simulink model "vegas01_4tap_1024ch_250mhz_r1"  (Simulink 7.6)
settings: Solver=ode45  StartTime=0.0  StopTime=128000  RelTol=1e-3
undefined symbols (not defined in the file): ROACH
libraries: xbsIndex_r4 x12350, monroe_library x84, xbsReplacements_r4 x36, xps_library x30, casper_library_misc x24, dspsigops x16
(part 9 of 103)

== system vegas01_4tap_1024ch_250mhz_r1/pfb_fir_real2/coeff_gen_2_5 ==
  sync : Inport
  Counter0 : xbsIndex_r4/Counter  cnt_type=Free Running  cnt_to=Inf  operation=Up  start_count=0  cnt_by_val=1  arith_type=Unsigned  n_bits=8  bin_pt=0  load_pin=off  rst=on  en=off  explicit_period=on  period=1  dbl_ovrd=off  use_behavioral_HDL=off  implementation=Fabric  block_type=counter
  Counter1 : xbsIndex_r4/Counter  cnt_type=Free Running  cnt_to=Inf  operation=Down  start_count=255  cnt_by_val=1  arith_type=Unsigned  n_bits=8  bin_pt=0  load_pin=off  rst=on  en=off  explicit_period=on  period=1  dbl_ovrd=off  use_behavioral_HDL=off  implementation=Fabric  block_type=counter
  coeff_ram_1 : xbsIndex_r4/Dual Port RAM  depth=256  initVector=[-3.90817859526566e-05 -0.00019581594613078 -0.000353262922406334 -0.000511505297702878 -0.000670626183556419 -0.000830709171084608 -0.00099183828144884 -0.00115409791582477 -0.00131757280489769 -0.00148234795789864 -0.00164850861119816 -0.00181614017647413 -0.00198532818847055 -0.00215615825236462 -0.00232871599075906 -0.00250308699031737 -0.00267935674805954 -0.00285761061733611 -0.00303793375349835 -0.003220411059283 -0.00340512712992955 -0.0035921661980487 -0.00378161207826056 -0.00397354811162116 -0.00416805710985634 -0.00436522129942181 -0.00456512226540876 -0.00476784089531378 -0.00497345732269304 -0.00518205087071952 -0.00539369999566348 -0.0056084822303152 -0.00582647412737009 -0.00604775120279601 -0.00627238787920226 -0.00650045742923075 -0.00673203191898883 -0.00696718215154433 -0.00720597761050248 -0.00744848640368526 -0.00769477520693305 -0.00794490920804915 -0.00819895205090707 -0.00845696577974117 -0.0087190107836408 -0.0089851457412682 -0.0092554275658206 -0.00952991135025664 -0.00980865031280764 -0.0100916957427936 -0.0103790969467645 -0.0106709011949873 -0.0109671536682976 -0.0112678974053384 -0.011573173250203 -0.011883019800505 -0.0121974733558934 -0.0125165678670334 -0.0128403348850725 -0.0131688035116118 -0.0135020003492023 -0.0138399494523852 -0.0141826722792961 -0.0145301876438517 -0.0148825116685399 -0.0152396577378301 -0.0156016364522244 -0.0159684555829678 -0.0163401200274364 -0.0167166317652216 -0.0170979898149293 -0.0174841901917123 -0.017875225865553 -0.0182710867203162 -0.0186717595135873 -0.0190772278373159 -0.0194874720792798 -0.0199024693853888 -0.0203221936228435 -0.0207466153441663 -0.0211757017521223 -0.0216094166655443 -0.0220477204860799 -0.0224905701658759 -0.0229379191762147 -0.02338971747712 -0.0238459114879449 -0.0243064440589593 -0.0247712544439492 -0.025240278273844 -0.0257134475313849 -0.0261906905268485 -0.0266719318748394 -0.0271570924721649 -0.0276460894768045 -0.0281388362879872 -0.0286352425273895 -0.0291352140214652 -0.0296386527849192 -0.0301454570053374 -0.030655521028983 -0.0311687353477706 -0.031684986587428 -0.0322041574968574 -0.0327261269387038 -0.0332507698811416 -0.0337779573908884 -0.0343075566274544 -0.0348394308386364 -0.0353734393572641 -0.0359094375992071 -0.0364472770626494 -0.0369868053286391 -0.0375278660629194 -0.0380702990190484 -0.0386139400428121 -0.0391586210779373 -0.0397041701731098 -0.040250411490302 -0.0407971653144147 -0.041344248064238 -0.0418914723047336 -0.0424386467606426 -0.0429855763314227 -0.0435320621075147 -0.044077901387944 -0.0446228876992558 -0.045166810815787 -0.0457094567812755 -0.0462506079318073 -0.0467900429201015 -0.0473275367411334 -0.0478628607590946 -0.0483957827356896 -0.0489260668597665 -0.0494534737782813 -0.0499777606285926 -0.050498681072084 -0.051015985329112 -0.051529420215275 -0.052038729179 -0.0525436523404422 -0.0530439265316944 -0.0535392853382986 -0.0540294591420561 -0.0545141751651301 -0.0549931575154334 -0.055466127233296 -0.0559328023394035 -0.0563928978840011 -0.0568461259973533 -0.0572921959414519 -0.0577308141629635 -0.0581616843474073 -0.0585845074745537 -0.058998981875033 -0.0594048032881459 -0.0598016649208627 -0.0601892575080014 -0.0605672693735733 -0.060935386493284 -0.0612932925581768 -0.0616406690394071 -0.0619771952541345 -0.0623025484325178 -0.0626164037858017 -0.0629184345754786 -0.0632083121835126 -0.0634857061836099 -0.0637502844135214 -0.0640017130483604 -0.0642396566749216 -0.0644637783669827 -0.064673739761574 -0.0648692011361979 -0.0650498214869818 -0.0652152586077456 -0.0653651691699669 -0.0654992088036259 -0.0656170321789096 -0.0657182930887587 -0.065802644532236 -0.0658697387986982 -0.0659192275527504 -0.0659507619199633 -0.0659639925733332 -0.0659585698204637 -0.0659341436914474 -0.0658903640274281 -0.0658268805698202 -0.0657433430501645 -0.0656394012805979 -0.0655147052449153 -0.0653689051901997 -0.0652016517189997 -0.0650125958820299 -0.0648013892713714 -0.0645676841141486 ...(+1158 chars)]  distributed_mem=Block RAM  init_a=0  init_b=0  rst_a=off  rst_b=off  en_a=off  en_b=off  latency=3  write_mode_A=No Read On Write  write_mode_B=No Read On Write  dbl_ovrd=off  optimize=Area  use_rpm=on  block_type=dpram
  coeff_ram_2 : xbsIndex_r4/Dual Port RAM  depth=256  initVector=[0.000528634489950224 0.00266729854995164 0.00484466034859744 0.00706084272688782 0.0093159621512389 0.0116101286431996 0.0139434457103661 0.0163160102785174 0.018727912624994 0.0211792363133421 0.0236700581292446 0.0262004480177607 0.0287704690218949 0.0313801772225176 0.0340296216796558 0.036718844375176 0.0394478801568792 0.0422167566840262 0.0450254943743139 0.0478741063523217 0.0507625983994457 0.0536909689053397 0.0566592088208814 0.0596673016126801 0.0627152232191451 0.0658029420081291 0.0689304187361667 0.0720976065093205 0.0753044507456532 0.0785508891393394 0.0818368516264341 0.0851622603523098 0.0885270296407792 0.0919310659649154 0.0953742679195836 0.0988565261956976 0.102377723556213 0.10593773481387 0.109536426810698 0.11317365839929 0.116849280425861 0.120563135715103 0.124315059056838 0.128104877194488 0.131932408815367 0.1357974645428 0.139699846930085 0.143639350456298 0.147615761523955 0.151628858458532 0.155678411509854 0.159764182855352 0.163885926605205 0.168043388809357 0.172236307466429 0.176464412534516 0.180727425943882 0.185025061611548 0.18935702545779 0.193723015424524 0.198122721495609 0.202555825719045 0.207022002231081 0.211520917282222 0.216052229265149 0.220615588744533 0.225210638488761 0.22983701350355 0.234494341067471 0.23918224076936 0.243900324547619 0.248648196731411 0.253425454083732 0.258231685846359 0.263066473786681 0.267929392246381 0.272820008191991 0.277737881267295 0.282682563847572 0.287653601095688 0.292650531020009 0.297672884534137 0.302720185518453 0.307791950883472 0.312887690634974 0.318006907940928 0.323149099200174 0.328313754112871 0.333500355752685 0.338708380640704 0.343937298821085 0.34918657393839 0.354455663316627 0.359744018039958 0.365051083035069 0.370376297155193 0.375719093265751 0.38107889833162 0.386455133505983 0.391847214220772 0.39725455027866 0.402676545946607 0.408112600050922 0.413562106073836 0.419024452251563 0.424499021673817 0.429985192384788 0.435482337485536 0.440989825237791 0.446507019169137 0.452033278179554 0.457567956649303 0.463110404548126 0.468659967545736 0.474215987123581 0.479777800687855 0.485344741683723 0.490916139710754 0.496491320639523 0.502069606729355 0.507650316747201 0.513232766087606 0.518816266893743 0.524400128179501 0.52998365595258 0.535566153338583 0.54114692070607 0.546725255792543 0.552300453831348 0.557871807679439 0.563438607946017 0.569000143121969 0.574555699710111 0.580104562356196 0.585646013980653 0.591179335911029 0.596703808015113 0.6022187088347 0.607723315719968 0.613216904964448 0.618698751940534 0.624168131235529 0.629624316788173 0.63506658202564 0.640494200000953 0.645906443530805 0.651302585333747 0.656681898168698 0.662043654973776 0.667387129005377 0.672711593977511 0.678016324201334 0.68330059472485 0.688563681472764 0.693804861386435 0.699023412563906 0.70421861439998 0.709389747726301 0.714536094951418 0.719656940200789 0.724751569456699 0.729819270698056 0.734859334040036 0.739871051873534 0.744853719004404 0.74980663279244 0.754729093290075 0.759620403380761 0.764479868916999 0.76930679885798 0.774100505406823 0.778860304147345 0.78358551418037 0.788275458259508 0.7929294629264 0.797546858645375 0.802126979937506 0.806669165514013 0.811172758409008 0.815637106111515 0.820061560696764 0.82444547895672 0.828788222529799 0.833089158029764 0.837347657173754 0.841563096909419 0.845734859541138 0.849862332855275 0.853944910244456 0.857981990830835 0.861972979588306 0.865917287463656 0.869814331496604 0.873663534938716 0.877464327371157 0.881216144821258 0.88491842987786 0.88857063180542 0.892172206656845 0.895722617385021 0.89922133395302 0.902667833442954 0.90606160016345 0.909402125755714 0.912688909298172 0.915921457409648 0.919099284351063 0.922221912125626 0.925288870577496 0.928299697488889 0.931253938675603 0.934151148080945 0.936990887868035 0.939772728510454 0.942496248881234 0.945161036340149 0.947766686819299 0.950312804906955 0.952799003929657 0.955224906032532 0.957590142257825 ...(+626 chars)]  distributed_mem=Block RAM  init_a=0  init_b=0  rst_a=off  rst_b=off  en_a=off  en_b=off  latency=3  write_mode_A=No Read On Write  write_mode_B=No Read On Write  dbl_ovrd=off  optimize=Area  use_rpm=on  block_type=dpram
  coeff_ram_3 : xbsIndex_r4/Dual Port RAM  depth=256  initVector=[0.999997585420204 0.999945862035735 0.999826625478213 0.999639894159387 0.999385696890974 0.999064072878614 0.998675071713642 0.99821875336269 0.997695188155105 0.997104456768201 0.996446650210344 0.99572186980187 0.994930227153842 0.99407184414466 0.993146852894515 0.992155395737707 0.991097625192822 0.98997370393079 0.988783804740809 0.987528110494175 0.986206814105993 0.984820118494805 0.983368236540129 0.98185139103793 0.980269814654024 0.978623749875434 0.976913448959704 0.975139173882195 0.973301196281355 0.971399797402003 0.969435268036615 0.967407908464641 0.965318028389877 0.963165946875873 0.960951992279441 0.958676502182232 0.956339823320431 0.953942311512578 0.951484331585526 0.94896625729856 0.946388471265699 0.943751364876193 0.941055338213235 0.938300799970914 0.935488167369428 0.932617866068564 0.929690330079492 0.926706001674867 0.923665331297283 0.920568777466085 0.917416806682576 0.914209893333624 0.91094851959372 0.907633175325473 0.904264357978609 0.900842572487458 0.897368331166986 0.89384215360738 0.89026456656721 0.88663610386521 0.882957306270688 0.879228721392593 0.875450903567282 0.87162441374499 0.867749819375054 0.863827694289899 0.859858618587827 0.855843178514634 0.851781966344081 0.847675580257249 0.843524624220809 0.839329707864236 0.835091446355994 0.830810460278722 0.826487375503459 0.822122823062921 0.817717439023884 0.81327186435868 0.808786744815852 0.80426273079 0.799700477190831 0.795100643311471 0.79046389269605 0.785790893006594 0.781082315889274 0.776338836840015 0.77156113506952 0.766749893367735 0.761905797967781 0.75702953840939 0.752121807401883 0.74718330068671 0.742214716899598 0.737216757432325 0.732190126294173 0.727135529973066 0.722053677296456 0.71694527929196 0.711811049047805 0.706651701573101 0.701467953657975 0.696260523733609 0.691030131732197 0.685777498946876 0.680503347891644 0.675208402161309 0.669893386291496 0.664559025618754 0.65920604614078 0.653835174376808 0.648447137228184 0.643042661839171 0.637622475457997 0.632187305298207 0.626737878400318 0.621274921493837 0.615799160859656 0.610311322192862 0.604812130466 0.5993023097928 0.593782583292427 0.588253672954258 0.582716299503241 0.577171182265841 0.571619039036634 0.566060585945546 0.560496537325794 0.554927605582544 0.549354501062321 0.543777931923198 0.538198604005794 0.532617220705113 0.527034482843242 0.52145108854295 0.515867733102212 0.510285108869672 0.504703905121097 0.499124807936828 0.493548500080263 0.487975660877405 0.482406966097486 0.476843087834706 0.47128469439111 0.465732450160622 0.460187015514271 0.454649046686618 0.449119195663434 0.443598110070618 0.438086433064416 0.432584803222934 0.42709385443898 0.421614215814265 0.41614651155497 0.410691360868709 0.405249377862908 0.399821171444622 0.394407345221811 0.389008497406085 0.383625220716957 0.378258102287602 0.37290772357216 0.367574660254585 0.362259482159064 0.356962753162037 0.351685031105807 0.34642686771378 0.341188808507344 0.335971392724402 0.330775153239571 0.325600616486067 0.32044830237929 0.315318724242122 0.310212388731944 0.305129795769404 0.300071438468922 0.295037803070971 0.290029368876126 0.285046608180895 0.280089986215362 0.275159961082616 0.27025698370001 0.265381497742243 0.260533939586272 0.255714738258069 0.250924315381225 0.246163085127416 0.241431454168725 0.23672982163184 0.232058579054126 0.227418110341571 0.222808791728628 0.218230991739939 0.213685071153951 0.209171382968434 0.204690272367895 0.200242076692889 0.195827125411242 0.191445740091166 0.187098234376291 0.182784913962595 0.178506076577238 0.174262011959301 0.170053001842433 0.165879319939389 0.161741231928475 0.157638995441885 0.153572860055929 0.149543067283158 0.145549850566363 0.141593435274461 0.137674038700255 0.133791870060063 0.129947130495211 0.126140013075378 0.1223707028038 0.118639376624309 0.114946203430207 0.111291344074969 0.107674951384763 0.10409717017277 0.100558137255318 0.0970579814697864 0.0935968236942969 0.090174776869171 ...(+608 chars)]  distributed_mem=Block RAM  init_a=0  init_b=0  rst_a=off  rst_b=off  en_a=off  en_b=off  latency=3  write_mode_A=No Read On Write  write_mode_B=No Read On Write  dbl_ovrd=off  optimize=Area  use_rpm=on  block_type=dpram
  coeff_ram_4 : xbsIndex_r4/Dual Port RAM  depth=256  initVector=[-0.000525881750074805 -0.00260537309808799 -0.00464651229172884 -0.0066494441433039 -0.00861431950540846 -0.0105412951944236 -0.0124305339129682 -0.0142822041713308 -0.0160964802079058 -0.0178735419086594 -0.0196135747256479 -0.021316769594617 -0.0229833228517039 -0.0246134361492695 -0.0262073163708863 -0.0277651755455068 -0.0292872307608388 -0.0307737040759537 -0.0322248224331538 -0.0336408175691244 -0.0350219259253981 -0.0363683885581564 -0.0376804510473964 -0.0389583634054881 -0.0402023799851507 -0.0414127593868716 -0.0425897643657997 -0.0437336617381345 -0.0448447222870422 -0.0459232206681237 -0.0469694353144624 -0.0479836483412778 -0.0489661454502136 -0.049917215833286 -0.0508371520765199 -0.0517262500632997 -0.052584808877463 -0.0534131307061621 -0.054211520742523 -0.0549802870881258 -0.0557197406553371 -0.0564301950695176 -0.0571119665711346 -0.0577653739178052 -0.0583907382862957 -0.0589883831745068 -0.0595586343034674 -0.060101819519367 -0.0606182686956506 -0.0611083136352037 -0.0615722879726526 -0.062010527076807 -0.06242336795327 -0.0628111491472417 -0.0631742106465414 -0.0635128937848755 -0.0638275411453737 -0.0641184964644211 -0.0643861045358101 -0.0646307111152371 -0.0648526628251682 -0.0650523070600987 -0.0652299918922314 -0.0653860659775954 -0.0655208784626324 -0.0656347788912711 -0.065728117112515 -0.0658012431885662 -0.0658545073035075 -0.0658882596725671 -0.0659028504519856 -0.0658986296495111 -0.0658759470355407 -0.0658351520549326 -0.0657765937395087 -0.0657006206212707 -0.0656075806463471 -0.0654978210896959 -0.0653716884705801 -0.065229528468837 -0.0650716858419616 -0.0648985043430223 -0.0647103266394287 -0.0645074942325704 -0.0642903473783444 -0.0640592250085901 -0.0638144646534491 -0.0635564023646674 -0.0632853726398575 -0.063001708347736 -0.062705740654355 -0.0623977989503416 -0.062078210779162 -0.061747301766426 -0.061405395550246 -0.0610528137126655 -0.0606898757121723 -0.0603168988173085 -0.0599341980413927 -0.0595420860783662 -0.0591408732397769 -0.058730867392913 -0.0583123739000985 -0.0578856955591619 -0.0574511325450902 -0.0570089823528784 -0.0565595397415847 -0.0561030966796028 -0.0556399422911595 -0.0551703628040478 -0.0546946414986045 -0.0542130586579401 -0.0537258915194295 -0.0532334142274719 -0.0527358977875257 -0.052233610021427 -0.0517268155239965 -0.0512157756209429 -0.050700748328066 -0.0501819883117671 -0.0496597468508707 -0.049134271799761 -0.0486058075528392 -0.0480745950103033 -0.0475408715452552 -0.0470048709721369 -0.0464668235164989 -0.0459269557861022 -0.0453854907433563 -0.0448426476790935 -0.0442986421876811 -0.0437536861434716 -0.0432079876785908 -0.0426617511620632 -0.0421151771802748 -0.041568462518771 -0.0410218001453889 -0.0404753791947211 -0.0399293849539095 -0.0393839988497661 -0.0388393984372169 -0.0382957573890664 -0.0377532454870785 -0.0372120286143683 -0.0366722687491021 -0.036134123959499 -0.0355977484001293 -0.0350632923095033 -0.034530902008945 -0.0340007199027441 -0.033472884479578 -0.0329475303151993 -0.0324247880763774 -0.0319047845260903 -0.031387642529954 -0.030873481063885 -0.0303624152229832 -0.0298545562316286 -0.0293500114547796 -0.0288488844104657 -0.028351274783461 -0.0278572784401305 -0.0273669874444371 -0.0268804900750966 -0.0263978708438723 -0.0259192105149939 -0.0254445861256901 -0.0249740710078223 -0.0245077348106065 -0.024045643524409 -0.0235878595056048 -0.0231344415024829 -0.0226854446821853 -0.0222409206586657 -0.0218009175216533 -0.021365479866606 -0.0209346488256395 -0.0205084620994157 -0.0200869539899756 -0.0196701554345008 -0.0192580940399877 -0.0188507941188172 -0.0184482767252055 -0.0180505596925173 -0.0176576576714256 -0.0172695821689009 -0.0168863415880124 -0.0165079412685241 -0.0161343835282672 -0.0157656677052719 -0.0154017902006412 -0.0150427445221464 -0.0146885213285283 -0.0143391084744838 -0.0139944910563209 -0.0136546514582619 -0.0133195693993767 -0.0129892219811274 -0.012663583735504 -0.0123426266737326 -0.0120263203355377 ...(+1148 chars)]  distributed_mem=Block RAM  init_a=0  init_b=0  rst_a=off  rst_b=off  en_a=off  en_b=off  latency=3  write_mode_A=No Read On Write  write_mode_B=No Read On Write  dbl_ovrd=off  optimize=Area  use_rpm=on  block_type=dpram
  const_din : xbsIndex_r4/Constant  arith_type=Signed (2's comp)  const=0  n_bits=18  bin_pt=17  explicit_period=on  period=1  equ=P=C  opselect=C  inp2=PCIN>>17  opr=+  inp1=P  carry=CIN  dbl_ovrd=off  block_type=constant
  const_we : xbsIndex_r4/Constant  arith_type=Boolean  const=0  n_bits=16  bin_pt=14  explicit_period=on  period=1  equ=P=C  opselect=C  inp2=PCIN>>17  opr=+  inp1=P  carry=CIN  dbl_ovrd=off  block_type=constant
  cram_lower : Subsystem
  cram_upper : Subsystem
  delay_a_addr1 : xbsIndex_r4/Delay  en=off  latency=2  dbl_ovrd=off  reg_retiming=off  block_type=delay
  delay_a_addr2 : xbsIndex_r4/Delay  en=off  latency=1  dbl_ovrd=off  reg_retiming=off  block_type=delay
  delay_b_addr3 : xbsIndex_r4/Delay  en=off  latency=1  dbl_ovrd=off  reg_retiming=off  block_type=delay
  delay_b_addr4 : xbsIndex_r4/Delay  en=off  latency=2  dbl_ovrd=off  reg_retiming=off  block_type=delay
  delay_sync : xbsIndex_r4/Delay  en=off  latency=3  dbl_ovrd=off  reg_retiming=off  block_type=delay
  sync_out : Outport
  coeff : Outport  Port=2
  coeff_rev : Outport  Port=3
  wires:
  sync/1 -> Counter1/1, Counter0/1, delay_sync/1
  Counter0/1 -> coeff_ram_4/1, coeff_ram_3/1, delay_a_addr2/1, delay_a_addr1/1
  const_din/1 -> coeff_ram_4/5, coeff_ram_4/2, coeff_ram_3/5, coeff_ram_3/2, coeff_ram_2/5, coeff_ram_2/2, coeff_ram_1/5, coeff_ram_1/2
  const_we/1 -> coeff_ram_4/6, coeff_ram_4/3, coeff_ram_3/6, coeff_ram_3/3, coeff_ram_2/6, coeff_ram_2/3, coeff_ram_1/6, coeff_ram_1/3
  Counter1/1 -> delay_b_addr4/1, delay_b_addr3/1, coeff_ram_2/4, coeff_ram_1/4
  cram_lower/1(out) -> coeff_rev/1
  cram_upper/1(out) -> coeff/1
  coeff_ram_1/2 -> cram_lower/4(in3)
  coeff_ram_2/2 -> cram_lower/3(in2)
  coeff_ram_3/2 -> cram_lower/2(in1)
  coeff_ram_4/2 -> cram_lower/1(in0)
  coeff_ram_4/1 -> cram_upper/4(in3)
  coeff_ram_3/1 -> cram_upper/3(in2)
  coeff_ram_2/1 -> cram_upper/2(in1)
  coeff_ram_1/1 -> cram_upper/1(in0)
  delay_b_addr4/1 -> coeff_ram_4/4
  delay_b_addr3/1 -> coeff_ram_3/4
  delay_a_addr2/1 -> coeff_ram_2/1
  delay_a_addr1/1 -> coeff_ram_1/1
  delay_sync/1 -> sync_out/1

== system vegas01_4tap_1024ch_250mhz_r1/pfb_fir_real2/coeff_gen_3_4 ==
  sync : Inport
  Counter0 : xbsIndex_r4/Counter  cnt_type=Free Running  cnt_to=Inf  operation=Up  start_count=0  cnt_by_val=1  arith_type=Unsigned  n_bits=8  bin_pt=0  load_pin=off  rst=on  en=off  explicit_period=on  period=1  dbl_ovrd=off  use_behavioral_HDL=off  implementation=Fabric  block_type=counter
  Counter1 : xbsIndex_r4/Counter  cnt_type=Free Running  cnt_to=Inf  operation=Down  start_count=255  cnt_by_val=1  arith_type=Unsigned  n_bits=8  bin_pt=0  load_pin=off  rst=on  en=off  explicit_period=on  period=1  dbl_ovrd=off  use_behavioral_HDL=off  implementation=Fabric  block_type=counter
  coeff_ram_1 : xbsIndex_r4/Dual Port RAM  depth=256  initVector=[-5.86373825914179e-05 -0.000215456147790523 -0.000372998021380911 -0.000531345655026345 -0.000690582222902466 -0.000850791372601726 -0.00101205717553319 -0.00117446407683278 -0.00133809684480029 -0.0015030405198794 -0.00166938036319721 -0.00183720180467995 -0.00200659039076204 -0.0021776317317052 -0.00235041144854523 -0.00252501511968397 -0.00270152822714364 -0.00288003610250207 -0.00306062387252614 -0.00324337640452213 -0.00342837825142082 -0.00361571359661625 -0.00380546619857627 -0.00399771933524413 -0.00419255574824945 -0.00439005758694802 -0.00459030635230934 -0.00479338284067104 -0.00499936708737985 -0.00520833831033822 -0.00542037485347644 -0.00563555413016958 -0.00585395256661942 -0.0060756455452207 -0.00630070734793194 -0.00652921109967067 -0.00676122871175293 -0.00699683082539746 -0.00723608675531428 -0.00747906443339831 -0.00772583035254768 -0.00797644951062753 -0.00823098535459909 -0.00848949972483472 -0.00875205279963877 -0.00901870303999501 -0.00928950713456065 -0.00956451994492721 -0.0098437944511688 -0.0101273816976976 -0.0104153307394476 -0.0107076885884055 -0.0110045001605102 -0.0113058082229405 -0.0116116533418109 -0.0119220738302957 -0.0122371056972012 -0.0125567825960062 -0.0128811357743903 -0.0132101940242697 -0.0135439836323603 -0.0138825283312874 -0.0142258492512617 -0.0145739648723409 -0.0149268909772952 -0.0152846406050976 -0.0156472240050563 -0.0160146485916082 -0.0163869188997935 -0.0167640365414281 -0.0171460001619934 -0.0175328053982617 -0.0179244448366743 -0.0183209079724907 -0.0187221811697278 -0.0191282476219039 -0.0195390873136075 -0.0199546769829069 -0.0203749900846166 -0.0207999967544396 -0.0212296637739995 -0.0216639545367806 -0.0221028290149906 -0.0225462437273637 -0.0229941517079172 -0.0234465024756791 -0.0239032420054004 -0.0243643126992679 -0.0248296533596316 -0.0252991991627612 -0.0257728816336456 -0.0262506286218495 -0.0267323642784407 -0.0272180090340002 -0.0277074795777305 -0.0282006888376717 -0.0286975459620406 -0.0291979563017022 -0.0297018213937885 -0.0302090389464727 -0.0307195028249126 -0.0312331030383722 -0.0317497257285335 -0.0322692531590073 -0.0327915637060538 -0.0333165318505221 -0.033844028171018 -0.0343739193383085 -0.0349060681109713 -0.0354403333322995 -0.0359765699284654 -0.0365146289079547 -0.0370543573622759 -0.0375955984679522 -0.0381381914898021 -0.038681971785515 -0.0392267708115271 -0.0397724161302025 -0.0403187314183254 -0.0408655364769064 -0.0414126472423082 -0.0419598757986934 -0.0425070303917987 -0.0430539154440375 -0.043600331570934 -0.0441460755988906 -0.0446909405842904 -0.0452347158339363 -0.0457771869268272 -0.0463181357372728 -0.0468573404593456 -0.047394575632671 -0.047929612169555 -0.0484622173834472 -0.0489921550187384 -0.0495191852818918 -0.0500430648739029 -0.0505635470240889 -0.0510803815252008 -0.051593314769858 -0.0521020897882994 -0.0526064462874469 -0.0531061206912774 -0.0536008461824974 -0.0540903527455154 -0.0545743672107048 -0.0550526132999523 -0.0555248116734847 -0.0559906799779658 -0.056449932895858 -0.0569022821960385 -0.0573474367856626 -0.0577851027632654 -0.058214983473093 -0.0586367795606527 -0.0590501890294728 -0.0594549072990618 -0.0598506272640558 -0.0602370393545433 -0.0606138315975561 -0.0609806896797129 -0.0613372970110058 -0.0616833347897144 -0.0620184820684369 -0.0623424158212223 -0.0626548110117918 -0.062955340662835 -0.0632436759263647 -0.0635194861551178 -0.0637824389749851 -0.0640322003584556 -0.0642684346990587 -0.064490804886788 -0.0646989723844908 -0.0648925973052045 -0.0650713384904255 -0.0652348535892895 -0.0653827991386482 -0.0655148306440217 -0.0656306026614101 -0.0657297688799434 -0.0658119822053517 -0.0658768948442361 -0.0659241583891191 -0.0659534239042566 -0.0659643420121883 -0.0659565629810087 -0.065929736812335 -0.0658835133299523 -0.0658175422691142 -0.0657314733664758 -0.0656249564506396 -0.065497641533289 -0.0653491789008882 -0.0651792192069268 -0.0649874135646831 -0.0647734136404861 -0.0645368717474501 ...(+1155 chars)]  distributed_mem=Block RAM  init_a=0  init_b=0  rst_a=off  rst_b=off  en_a=off  en_b=off  latency=3  write_mode_A=No Read On Write  write_mode_B=No Read On Write  dbl_ovrd=off  optimize=Area  use_rpm=on  block_type=dpram
  coeff_ram_2 : xbsIndex_r4/Dual Port RAM  depth=256  initVector=[0.000793855568781102 0.00293734991211368 0.00511955769539444 0.00734060096668454 0.00960059539061112 0.0118996501782322 0.0142378680181029 0.016615345008565 0.0190321705912827 0.0214884274860464 0.0239841916268662 0.0265195320993763 0.0290945110795724 0.0317091837739016 0.0343635983607266 0.0370577959331841 0.0397918104434568 0.04256566864848 0.0453793900570998 0.0482329868787049 0.051126463973348 0.0540598188033769 0.0570330413865915 0.0600461142509466 0.0630990123908156 0.066191703224833 0.0693241465553323 0.072496294529395 0.0757080916015266 0.0789594744979745 0.0822503721827049 0.0855807058250493 0.0889503887690387 0.0923593265044371 0.095807416639488 0.0992945488753877 0.102820604982496 0.1063854587783 0.109988976107135 0.113631014821687 0.117311424766273 0.121030047761919 0.124786717593239 0.128581259997137 0.132413492653321 0.136283225176654 0.140190259111347 0.144134387926999 0.148115397016481 0.152133063695701 0.156187157205215 0.16027743871373 0.164403661323472 0.168565570077445 0.172762901968574 0.176995385950742 0.18126274295172 0.185564685887996 0.189900919681506 0.19427114127826 0.198675039668881 0.203112295911043 0.207582583153816 0.21208556666391 0.216620903853836 0.221188244311956 0.225787229834444 0.230417494459145 0.235078664501327 0.239770358591334 0.244492187714121 0.249243755250686 0.254024657021376 0.258834481331072 0.263672809016247 0.268539213493888 0.273433260812281 0.278354509703641 0.283302511638591 0.28827681088248 0.293276944553524 0.29830244268277 0.303352828275865 0.308427617376632 0.31352631913243 0.318648435861293 0.323793463120839 0.328960889778932 0.334150198086085 0.339360863749602 0.344592356009424 0.349844137715687 0.355115665407971 0.360406389396214 0.365715753843293 0.371043196849245 0.376388150537122 0.381750041140444 0.387128289092261 0.392522309115784 0.397931510316576 0.403355296276285 0.408793065147898 0.414244209752503 0.419708117677526 0.42518417137644 0.430671748269917 0.436170220848394 0.441678956776052 0.447197318996171 0.452724665837839 0.458260351124004 0.463803724280834 0.469354130448373 0.474910910592456 0.480473401617876 0.486040936482762 0.491612844314159 0.497188450524771 0.502767076930856 0.508348041871229 0.513930660327374 0.519514244044604 0.525098101654283 0.530681538797043 0.536263858246998 0.541844360036917 0.547422341584325 0.552997097818512 0.558567921308413 0.564134102391344 0.569694929302543 0.575249688305515 0.580797663823129 0.586338138569444 0.591870393682245 0.597393708856244 0.602907362476923 0.608410631754986 0.613902792861399 0.61938312106297 0.624850890858456 0.630305376115151 0.635745850205934 0.641171586146744 0.646581856734442 0.651975934685042 0.657353092772267 0.662712603966406 0.668053741573435 0.673375779374372 0.678677991764833 0.683959653894763 0.689220041808292 0.694458432583704 0.699674104473478 0.704866337044359 0.710034411317448 0.715177609908251 0.72029521716668 0.725386519316956 0.730450804597387 0.735487363399991 0.740495488409925 0.745474474744689 0.750423620093078 0.755342224853842 0.760229592274024 0.76508502858694 0.769907843149779 0.774697348580773 0.779452860895924 0.784173699645239 0.788859188048447 0.793508653130172 0.79812142585452 0.802696841259047 0.807234238588094 0.811732961425428 0.816192357826184 0.820611780448065 0.824990586681763 0.829328138780581 0.83362380398922 0.837876954671699 0.842086968438377 0.846253228272049 0.850375122653088 0.854452045683591 0.858483397210515 0.862468582947766 0.866407014597203 0.870298109968547 0.874141293098149 0.877935994366595 0.88168165061512 0.885377705260805 0.889023608410519 0.892618816973592 0.896162794773185 0.899655012656325 0.90309494860259 0.906482087831407 0.909815922907943 0.913095953847561 0.916321688218814 0.919492641244963 0.922608335903972 0.925668303026978 0.928672081395204 0.931619217835282 0.934509267312983 0.937341793025309 0.940116366490941 0.942832567639016 0.945489984896204 0.948088215272075 0.950626864442732 0.953105546832681 0.955523885694932 0.9578815131893 ...(+626 chars)]  distributed_mem=Block RAM  init_a=0  init_b=0  rst_a=off  rst_b=off  en_a=off  en_b=off  latency=3  write_mode_A=No Read On Write  write_mode_B=No Read On Write  dbl_ovrd=off  optimize=Area  use_rpm=on  block_type=dpram
  coeff_ram_3 : xbsIndex_r4/Dual Port RAM  depth=256  initVector=[0.999994812497531 0.999934649315113 0.999806974692208 0.999611808340819 0.999349180372279 0.999019131290932 0.998621711985642 0.998156983719109 0.99762501811503 0.997025897143069 0.996359713101675 0.995626568598733 0.994826576530044 0.993959860055675 0.993026552574138 0.992026797694442 0.990960749206007 0.989828571046454 0.988630437267265 0.987366531997347 0.986037049404487 0.984642193654713 0.983182178869577 0.981657229081366 0.980067578186247 0.978413469895374 0.976695157683941 0.974912904738229 0.973066983900625 0.971157677612657 0.96918527785603 0.967150086091704 0.965052413197015 0.962892579400849 0.9606709142169 0.95838775637502 0.956043453750677 0.953638363292534 0.951172850948186 0.948647291588047 0.946062068927424 0.94341757544679 0.940714212310279 0.937952389282417 0.935132524643115 0.932255045100944 0.929320385704707 0.926328989753344 0.923281308704171 0.920177802079496 0.917018937371619 0.913805189946252 0.910537042944371 0.907214987182531 0.903839521051669 0.90041115041441 0.896930388500917 0.893397755803297 0.889813779968586 0.886178995690362 0.88249394459898 0.878759175150481 0.87497524251419 0.871142708459034 0.867262141238604 0.863334115474993 0.85935921204144 0.855338017943796 0.851271126200856 0.847159135723579 0.843002651193223 0.83880228293843 0.834558646811279 0.83027236406236 0.825944061214867 0.821574369937774 0.817163926918096 0.81271337373228 0.808223356716763 0.803694526837708 0.799127539559968 0.7945230547153 0.789881736369862 0.785204252691029 0.780491275813551 0.775743481705095 0.770961550031191 0.766146164019636 0.761298010324352 0.756417778888776 0.751506162808768 0.74656385819511 0.741591564035602 0.736589982056795 0.731559816585398 0.726501774409391 0.721416564638871 0.716304898566669 0.711167489528765 0.706005052764548 0.700818305276932 0.695607965692387 0.690374754120884 0.685119392015829 0.679842602033979 0.674545107895397 0.669227634243474 0.663890906505042 0.658535650750622 0.653162593554832 0.647772461856991 0.642365982821949 0.636943883701178 0.631506891694151 0.626055733810047 0.620591136729813 0.61511382666861 0.609624529238677 0.604123969312646 0.598612870887343 0.593091956948089 0.587561949333563 0.582023568601217 0.576477533893316 0.570924562803598 0.56536537124461 0.559800673315729 0.554231181171917 0.548657604893226 0.543080652355085 0.537501029099403 0.53191943820651 0.526336580167975 0.520753152760311 0.515169850919615 0.50958736661716 0.504006388735964 0.498427602948374 0.492851691594681 0.487279333562795 0.481711204169014 0.476147975039901 0.470590313995303 0.465038884932535 0.459494347711752 0.453957358042535 0.448428567371718 0.442908622772472 0.437398166834681 0.431897837556619 0.426408268237969 0.420930087374181 0.415463918552222 0.410010380347709 0.404570086223473 0.39914364442955 0.393731657904643 0.388334724179058 0.382953435279135 0.377588377633211 0.372240131979102 0.366909273273159 0.361596370600881 0.35630198708913 0.351026679819948 0.345770999746003 0.340535491607668 0.335320693851764 0.330127138551971 0.324955351330922 0.319805851284001 0.314679150904859 0.309575756012646 0.304496165680994 0.299440872168748 0.294410360852464 0.28940511016068 0.284425591509976 0.279472269242834 0.274545600567304 0.269646035498482 0.264774016801823 0.259929979938285 0.255114353011316 0.250327556715688 0.245570004288195 0.240842101460215 0.236144246412134 0.231476829729653 0.226840234361979 0.222234835581892 0.21766100094771 0.213119090267143 0.208609455563046 0.20413244104107 0.199688383059216 0.195277610099287 0.19090044274025 0.186557193633502 0.182248167480034 0.177973661009513 0.173733962961252 0.169529354067092 0.165360107036185 0.161226486541674 0.15712874920926 0.153067143607678 0.149041910241047 0.14505328154311 0.141101481873355 0.137186727515006 0.133309226674887 0.129469179485146 0.125666778006837 0.121902206235346 0.118175640107666 0.114487247511497 0.110837188296181 0.107225614285441 0.103652669291933 0.100118489133601 0.0966232016518021 0.0931669267312255 ...(+627 chars)]  distributed_mem=Block RAM  init_a=0  init_b=0  rst_a=off  rst_b=off  en_a=off  en_b=off  latency=3  write_mode_A=No Read On Write  write_mode_B=No Read On Write  dbl_ovrd=off  optimize=Area  use_rpm=on  block_type=dpram
  coeff_ram_4 : xbsIndex_r4/Dual Port RAM  depth=256  initVector=[-0.000787920351828344 -0.00286260996943633 -0.00489896520129326 -0.00689713161888919 -0.00885726082449819 -0.0107795103745431 -0.0126640437019187 -0.0145110300372983 -0.0163206443294465 -0.0180930671645657 -0.0198284846846987 -0.0215270885052133 -0.0231890756313952 -0.0248146483741729 -0.0264040142650019 -0.0279573859699323 -0.0294749812028871 -0.0309570226381763 -0.0324037378222731 -0.0338153590848793 -0.0351921234493043 -0.036534272542187 -0.0378420525025839 -0.0391157138904531 -0.0403555115945585 -0.0415617047398231 -0.042734556594156 -0.0438743344747823 -0.0449813096541012 -0.0460557572651 -0.0470979562063509 -0.0481081890466181 -0.0490867419291014 -0.0500339044753439 -0.0509499696888316 -0.0518352338583101 -0.0526899964608479 -0.0535145600646716 -0.0543092302318 -0.0550743154205058 -0.0558101268876299 -0.0565169785907762 -0.0571951870904141 -0.0578450714519141 -0.0584669531475448 -0.0590611559584568 -0.0596280058766808 -0.0601678310071659 -0.0606809614698843 -0.0611677293020293 -0.0616284683603321 -0.0620635142235239 -0.0624732040949686 -0.0628578767054923 -0.0632178722164352 -0.0635535321229509 -0.063865199157579 -0.0641532171941154 -0.0644179311518061 -0.0646596868998882 -0.0648788311625045 -0.0650757114240131 -0.0652506758347198 -0.0654040731170541 -0.065536252472215 -0.0656475634873088 -0.0657383560430019 -0.0658089802217137 -0.0658597862163704 -0.0658911242397428 -0.0659033444343921 -0.0658967967832431 -0.0658718310208095 -0.0658287965450905 -0.0657680423301619 -0.0656899168394816 -0.0655947679399311 -0.0654829428166129 -0.0653547878884241 -0.0652106487244269 -0.0650508699610347 -0.0648757952200333 -0.0646857670274563 -0.0644811267333347 -0.0642622144323359 -0.0640293688853139 -0.0637829274417857 -0.0635232259633528 -0.0632505987480838 -0.0629653784558762 -0.0626678960348121 -0.0623584806485253 -0.0620374596045948 -0.0617051582839797 -0.0613619000715118 -0.0610080062874585 -0.0606437961201723 -0.0602695865598394 -0.0598856923333414 -0.0594924258402432 -0.0590900970899198 -0.0586790136398347 -0.0582594805349814 -0.0578318002484996 -0.0573962726234782 -0.0569531948159544 -0.0565028612391215 -0.0560455635087529 -0.0555815903898541 -0.0551112277445508 -0.0546347584812225 -0.0541524625048896 -0.0536646166688625 -0.0531714947276601 -0.0526733672912054 -0.0521705017803039 -0.051663162383413 -0.051151610014707 -0.0506361022734436 -0.0501168934046382 -0.0495942342610489 -0.0490683722664788 -0.0485395513803979 -0.0480080120638888 -0.0474739912469198 -0.0469377222969473 -0.0463994349888512 -0.0458593554762035 -0.0453177062638732 -0.0447747061819681 -0.0442305703611143 -0.0436855102090731 -0.0431397333886967 -0.0425934437972209 -0.0420468415468947 -0.0415001229469451 -0.0409534804868759 -0.040407102821098 -0.0398611747548894 -0.0393158772316811 -0.0387713873216666 -0.0382278782117312 -0.0376855191966968 -0.0371444756718779 -0.0366049091269439 -0.0360669771410839 -0.035530833379466 -0.0349966275909875 -0.0344645056073088 -0.0339346093431641 -0.0334070767979427 -0.0328820420585328 -0.0323596353034207 -0.0318399828080369 -0.0313232069513411 -0.0308094262236367 -0.0302987552356068 -0.0297913047285612 -0.0292871815858849 -0.0287864888456782 -0.0282893257145784 -0.0277957875827514 -0.0273059660400422 -0.0268199488932742 -0.0263378201846839 -0.0258596602114802 -0.0253855455465156 -0.024915549060056 -0.0244497399426378 -0.0239881837289974 -0.0235309423230611 -0.0230780740239801 -0.0226296335531983 -0.0221856720825369 -0.0217462372632829 -0.0213113732562652 -0.0208811207629043 -0.0204555170572198 -0.02003459601878 -0.0196183881665788 -0.019206920693822 -0.0188002175036087 -0.0183982992454906 -0.0180011833528919 -0.0176088840813737 -0.0172214125477258 -0.0168387767698682 -0.0164609817075447 -0.0160880293037919 -0.0157199185271648 -0.015356645414701 -0.0149982031156059 -0.0146445819356409 -0.0142957693821939 -0.013951750210017 -0.0136125064676089 -0.0132780175442264 -0.012948260217503 -0.012623208701658 -0.0123028346962748 ...(+1170 chars)]  distributed_mem=Block RAM  init_a=0  init_b=0  rst_a=off  rst_b=off  en_a=off  en_b=off  latency=3  write_mode_A=No Read On Write  write_mode_B=No Read On Write  dbl_ovrd=off  optimize=Area  use_rpm=on  block_type=dpram
  const_din : xbsIndex_r4/Constant  arith_type=Signed (2's comp)  const=0  n_bits=18  bin_pt=17  explicit_period=on  period=1  equ=P=C  opselect=C  inp2=PCIN>>17  opr=+  inp1=P  carry=CIN  dbl_ovrd=off  block_type=constant
  const_we : xbsIndex_r4/Constant  arith_type=Boolean  const=0  n_bits=16  bin_pt=14  explicit_period=on  period=1  equ=P=C  opselect=C  inp2=PCIN>>17  opr=+  inp1=P  carry=CIN  dbl_ovrd=off  block_type=constant
  cram_lower : Subsystem
  cram_upper : Subsystem
  delay_a_addr1 : xbsIndex_r4/Delay  en=off  latency=2  dbl_ovrd=off  reg_retiming=off  block_type=delay
  delay_a_addr2 : xbsIndex_r4/Delay  en=off  latency=1  dbl_ovrd=off  reg_retiming=off  block_type=delay
  delay_b_addr3 : xbsIndex_r4/Delay  en=off  latency=1  dbl_ovrd=off  reg_retiming=off  block_type=delay
  delay_b_addr4 : xbsIndex_r4/Delay  en=off  latency=2  dbl_ovrd=off  reg_retiming=off  block_type=delay
  delay_sync : xbsIndex_r4/Delay  en=off  latency=3  dbl_ovrd=off  reg_retiming=off  block_type=delay
  sync_out : Outport
  coeff : Outport  Port=2
  coeff_rev : Outport  Port=3
  wires:
  sync/1 -> Counter1/1, Counter0/1, delay_sync/1
  Counter0/1 -> coeff_ram_4/1, coeff_ram_3/1, delay_a_addr2/1, delay_a_addr1/1
  const_din/1 -> coeff_ram_4/5, coeff_ram_4/2, coeff_ram_3/5, coeff_ram_3/2, coeff_ram_2/5, coeff_ram_2/2, coeff_ram_1/5, coeff_ram_1/2
  const_we/1 -> coeff_ram_4/6, coeff_ram_4/3, coeff_ram_3/6, coeff_ram_3/3, coeff_ram_2/6, coeff_ram_2/3, coeff_ram_1/6, coeff_ram_1/3
  Counter1/1 -> delay_b_addr4/1, delay_b_addr3/1, coeff_ram_2/4, coeff_ram_1/4
  cram_lower/1(out) -> coeff_rev/1
  cram_upper/1(out) -> coeff/1
  coeff_ram_1/2 -> cram_lower/4(in3)
  coeff_ram_2/2 -> cram_lower/3(in2)
  coeff_ram_3/2 -> cram_lower/2(in1)
  coeff_ram_4/2 -> cram_lower/1(in0)
  coeff_ram_4/1 -> cram_upper/4(in3)
  coeff_ram_3/1 -> cram_upper/3(in2)
  coeff_ram_2/1 -> cram_upper/2(in1)
  coeff_ram_1/1 -> cram_upper/1(in0)
  delay_b_addr4/1 -> coeff_ram_4/4
  delay_b_addr3/1 -> coeff_ram_3/4
  delay_a_addr2/1 -> coeff_ram_2/1
  delay_a_addr1/1 -> coeff_ram_1/1
  delay_sync/1 -> sync_out/1

== system vegas01_4tap_1024ch_250mhz_r1/pfb_fir_real2/multi_delay_bram_fast_0 ==
  in0 : Inport
  in1 : Inport  Port=2
  in2 : Inport  Port=3
  in3 : Inport  Port=4
  in4 : Inport  Port=5
  in5 : Inport  Port=6
  Concat2_1 : xbsIndex_r4/Concat  num_inputs=2  dbl_ovrd=off  block_type=concat
  cram : Subsystem
  cram_all : Subsystem
  double_delay_bram_fast1 : monroe_library/double_delay_bram_fast  SystemSampleTime=-1  delay_len=256  bram_latency=2  register_counter=1
  double_delay_bram_fast2 : monroe_library/delay_bram_fast  SystemSampleTime=-1  delay_len=256  bram_latency=2  memory_type=Block RAM  register_counter=1
  sliceA_1 : xbsIndex_r4/Slice  nbits=18  boolean_output=off  mode=Upper Bit Location + Width  bit1=-0  base1=MSB of Input  bit0=-0  base0=MSB of Input  dbl_ovrd=off  block_type=slice
  sliceB_1 : xbsIndex_r4/Slice  nbits=18  boolean_output=off  mode=Upper Bit Location + Width  bit1=-18  base1=MSB of Input  bit0=-18  base0=MSB of Input  dbl_ovrd=off  block_type=slice
  slice_last : xbsIndex_r4/Slice  nbits=12  boolean_output=off  mode=Lower Bit Location + Width  bit1=0  base1=LSB of Input  bit0=0  base0=LSB of Input  dbl_ovrd=off  block_type=slice
  uncram : Subsystem
  out0 : Outport
  out1 : Outport  Port=2
  out2 : Outport  Port=3
  out3 : Outport  Port=4
  out4 : Outport  Port=5
  out5 : Outport  Port=6
  wires:
  cram/1(out) -> slice_last/1, sliceB_1/1, sliceA_1/1
  uncram/6(out5) -> out5/1
  uncram/5(out4) -> out4/1
  uncram/4(out3) -> out3/1
  uncram/3(out2) -> out2/1
  uncram/2(out1) -> out1/1
  uncram/1(out0) -> out0/1
  cram_all/1(out) -> uncram/1(in)
  double_delay_bram_fast2/1 -> cram_all/2(in1)
  Concat2_1/1 -> cram_all/1(in0)
  slice_last/1 -> double_delay_bram_fast2/1
  double_delay_bram_fast1/2 -> Concat2_1/2
  double_delay_bram_fast1/1 -> Concat2_1/1
  sliceB_1/1 -> double_delay_bram_fast1/2
  sliceA_1/1 -> double_delay_bram_fast1/1
  in5/1 -> cram/6(in5)
  in4/1 -> cram/5(in4)
  in3/1 -> cram/4(in3)
  in2/1 -> cram/3(in2)
  in1/1 -> cram/2(in1)
  in0/1 -> cram/1(in0)
